simulink model "SerialPractice"  (R2017a)
settings: Solver=FixedStepAuto  StartTime=0.0  StopTime=10  FixedStep=0.01  MinStep=0.001  RelTol=1e-3  EnableMultiTasking=on
model workspace (MAT data in the model file):
  A = -0.291988550171
  B = -3.35930459163
  BladeGeometry = [0.0525  0.0575  0.0625  0.0675  0.0725  0.0775  0.0825  0.0875  0.0925  0.0975  0.1025  0.1075  0.1125  0.1175  0.1225  0.1275  0.1325  0.1375  0.1425  0.1475  0.1525  0.1575  0.1625  0.1675  0.1725  0.1775  0.1825  0.1875  0.1925  0.1975  0.2025  0.2075  0.2125  0.2175; 0.08  0.0765  0.073  0.0695  0.066  0.0625  0.059  0.0555  0.052  0.04975  0.0475  0.04525  0.043  0.0402857  0.0387143  0.0371429  0.0355714  0.034  0.0333333  0.0326667  0.032  0.0314615  0.0309231  0.0303846  0.0298462  0.0293077  0.0287692  0.0282308  0.0276923  0.0271538  0.0266154  0.0260769  0.0255385  0.025; 0.488692190558  0.471238898038  0.453785605519  0.436332312999  0.418879020479  0.401425727959  0.383972435439  0.366519142919  0.349065850399  0.331612557879  0.314159265359  0.296705972839  0.279252680319  0.270526034059  0.261799387799  0.244346095279  0.226892802759  0.209439510239  0.191986217719  0.174532925199  0.157079632679  0.157079632679  0.13962634016  0.13962634016  0.1308996939  0.12217304764  0.12217304764  0.10471975512  0.10471975512  0.10471975512  0.0872664625997  0.0872664625997  0.0698131700798  0.0698131700798]
  BladeRadius = Simulink.Parameter
  BladeRadius.Value = 0.225
  BladeRadius.Description = 'Radius of wind turbine blades'
  BladeRadius.DataType = 'auto'
  BladeRadius.DocUnits = 'm'
  BladeRadius.Dimensions = [1 1]
  C = 1
  CMCoeff = [0.0123861263102 0.0100869558121 0.00395957088672 0.0120046459416 0.000145393148265 0.000524554028707 -0.00381348366885 -0.000510658981973 -0.000304808013498 2.16037945221e-05 0.000267649913084 4.61550112894e-05 9.1778058191e-06 -1.06753844495e-05 -3.61000286217e-07]
  Cd = 0
  CpCoeff = [0.0934076671766 -0.182695655179 -0.0130608968061 0.168650159118 0.0246505329395 0.00117759523912 -0.0369025055261 -0.00816552386632 -0.000573147306806 5.84997495063e-05 0.00238658647448 0.000659038696901 -7.31985706862e-05 -3.57393703765e-05 -1.38327249044e-06]
  CpMat = [0.00867983704985 0.0104119952175 0.0123710198164 0.0145730804094 0.0170242990451 0.0197435241026 0.0227463845937 0.0260322039195 0.0296166959289 0.0335240991161 0.0377450541312 0.0423117873146 ... (4998 elements, 98x51)]
  CpMat_Iter4 = [-0.0174922752096 -0.0192000701213 -0.0208598028222 -0.0224761244863 -0.0240370794106 -0.0255486645229 -0.0270020954202 -0.0283906118498 -0.0297102509597 -0.0309617439636 -0.0321423489123 -0.0332305675829 ... (1116 elements, 31x36)]
  CqMat = [0.00950017223156 0.0100359591617 0.0106270141471 0.0112699479611 0.0119664102911 0.012716418653 0.0135224725245 0.0143788698852 0.0152889983241 0.0162542310373 0.017269427342 0.0183409321549 ... (4998 elements, 98x51)]
  CqMat_Iter4 = [-0.00651761334335 -0.00659827180546 -0.00666156814481 -0.00671038770655 -0.00674205252964 -0.00676213602267 -0.00676868409106 -0.00675993762446 -0.00673707609481 -0.00670413785211 -0.00666096044769 -0.00660422169135 ... (1116 elements, 31x36)]
  CtMat = [0.288467055178 0.29651503174 0.305320968805 0.314902207207 0.325269269239 0.336435131701 0.348427518089 0.36126603808 0.374993913486 0.389643061676 0.405260174906 0.421894254361 ... (4998 elements, 98x51)]
  CtMat_Iter4 = [0.270159937237 0.280676295654 0.292081972796 0.304364398908 0.317529220148 0.331577864133 0.346520948014 0.362369170866 0.379136481365 0.396833726898 0.415486832803 0.435128972133 ... (1116 elements, 31x36)]
  D = 0
  F = 8.99888284546
  G1 = 3.42440748358
  G2 = 0.707106781187
  GenPowerMat = [2.1012  1.98778255814  1.98947398844  1.833  1.70067971429  1.60026934097  1.384  1.0602; 9.4178  9.28771731844  8.83061432665  8.22752322946  7.62277971429  7.15313467049  6.01480738636  4.6053; 21.4169168091  21.0754826816  20.2670740984  18.7315883853  17.5955201987  16.3692763533  13.7873926136  10.6428; 35.1462940199  34.5144  33.2681888525  31.0593408638  29.3015798013  26.962289701  23.0149870432  17.6614; 48.8871910299  47.9983864865  49.251115894  45.8618066667  43.6489896806  40.1456687919  34.2611  26.4765; 67.2083870432  65.9770351351  70.6018443709  69.518850495  66.5248619165  60.9904074074  51.834  40.6659472362]
  GenTorqMat = [0.0501624549637  0.047454812988  0.0474951929119  0.0437596516031  0.0406007374717  0.0382036163842  0.0330405661859  0.0253104105999; 0.119911153844  0.118254889702  0.11243487365  0.10475607931  0.0970562457303  0.0910765393128  0.0765829061828  0.0586365007537; 0.185924082325  0.182960031645  0.17594209226  0.162612266372  0.152749855412  0.142104613443  0.11969081928  0.0923920488185; 0.239730275341  0.235420172908  0.226919858725  0.211853469756  0.199863911391  0.183907786414  0.156983526562  0.120467104797; 0.274610756692  0.269618134227  0.276655007553  0.257616466946  0.245186557716  0.225507587011  0.192452591321  0.148724677086; 0.305615629004  0.300016322066  0.321046643515  0.316121962696  0.302507446072  0.277340709145  0.235703923435  0.184919614804]
  J = Simulink.Parameter
  J.Value = 0.01
  J.Description = 'Resistance Inertia (0.2708)'
  J.DataType = 'auto'
  J.DocUnits = 'kg*m^2'
  J.Dimensions = [1 1]
  K = -0.496937064767
  Kd = Simulink.Parameter
  Kd.Value = 3
  Kd.DataType = 'auto'
  Kd.Dimensions = [1 1]
  Ki = Simulink.Parameter
  Ki.Value = 3
  Ki.DataType = 'auto'
  Ki.Dimensions = [1 1]
  Kp = Simulink.Parameter
  Kp.Value = 4
  Kp.DataType = 'auto'
  Kp.Dimensions = [1 1]
  Kt = Simulink.Parameter
  Kt.Value = 0.045
  Kt.Description = 'Torque Constant
    | '
  Kt.DataType = 'auto'
  Kt.DocUnits = 'N*m/A'
  Kt.Dimensions = [1 1]
  MaxPitch = Simulink.Parameter
  MaxPitch.Value = 30
  MaxPitch.Description = 'Maximum pitch angle'
  MaxPitch.DataType = 'auto'
  MaxPitch.DocUnits = 'deg'
  MaxPitch.Dimensions = [1 1]
  MaxPitchRate = Simulink.Parameter
  MaxPitchRate.Value = 150
  MaxPitchRate.Description = 'Maximum pitch rate'
  MaxPitchRate.DataType = 'auto'
  MaxPitchRate.DocUnits = 'deg/s'
  MaxPitchRate.Dimensions = [1 1]
  MinPitch = Simulink.Parameter
  MinPitch.Value = -10
  MinPitch.Description = 'Minimum pitch angle'
  MinPitch.DataType = 'auto'
  MinPitch.Dimensions = [1 1]
  N = Simulink.Parameter
  N.Value = 3
  N.Description = 'Number of blades'
  N.DataType = 'auto'
  N.Dimensions = [1 1]
  Ns = Simulink.Parameter
  Ns.Value = 2300
  Ns.Description = 'Generator sychronous speed'
  Ns.DataType = 'auto'
  Ns.DocUnits = 'rpm'
  Ns.Dimensions = [1 1]
  Param = Simulink.Parameter
  Param.DataType = 'auto'
  Param.Dimensions = [0 0]
  Param1 = Simulink.Parameter
  Param1.DataType = 'auto'
  Param1.Dimensions = [0 0]
  PowerMat = [0 0.000462072097155 0.000925921899779 0.00139155621296 0.00185898187069 0.00232820573596 0.00279923470096 0.00327207568717 0.00374673564551 0.00422322155651 0.00470154043042 0.00518169930735 ... (3512982 elements, 6666x17x31)]
  Qcog = Simulink.Parameter
  Qcog.Value = 0.01
  Qcog.Description = 'Cogging Torque'
  Qcog.DataType = 'auto'
  Qcog.DocUnits = 'N*m'
  Qcog.Dimensions = [1 1]
  R1 = Simulink.Parameter
  R1.Value = 4000
  R1.Description = 'Resistance of Divider_1'
  R1.DataType = 'auto'
  R1.DocUnits = 'Ω'
  R1.Dimensions = [1 1]
  R11 = Simulink.Parameter
  R11.Value = 1000
  R11.Description = 'Resistance of Divider_1.1'
  R11.DataType = 'auto'
  R11.DocUnits = 'Ω'
  R11.Dimensions = [1 1]
  R1_g = Simulink.Parameter
  R1_g.Value = 0.461
  R1_g.Description = 'A resistance in the generator'
  R1_g.DataType = 'auto'
  R1_g.DocUnits = 'Ω'
  R1_g.Dimensions = [1 1]
  R2 = Simulink.Parameter
  R2.Value = 4000
  R2.Description = 'Resistance of Divider_2'
  R2.DataType = 'auto'
  R2.DocUnits = 'Ω'
  R2.Dimensions = [1 1]
  R21 = Simulink.Parameter
  R21.Value = 1000
  R21.Description = 'Resistance of Divider_2.1'
  R21.DataType = 'auto'
  R21.DocUnits = 'Ω'
  R21.Dimensions = [1 1]
  R2_g = Simulink.Parameter
  R2_g.Value = 0.258
  R2_g.Description = 'A resistance in the generator'
  R2_g.DataType = 'auto'
  R2_g.DocUnits = 'Ω'
  R2_g.Dimensions = [1 1]
  R3 = Simulink.Parameter
  R3.Value = 4000
  R3.Description = 'Resistance of Divider_3'
  R3.DataType = 'auto'
  R3.DocUnits = 'Ω'
  R3.Dimensions = [1 1]
  R31 = Simulink.Parameter
  R31.Value = 1000
  R31.Description = 'Resistance of Divider_3.1'
  R31.DataType = 'auto'
  R31.DocUnits = 'Ω'
  R31.Dimensions = [1 1]
  R4 = Simulink.Parameter
  R4.Value = 4000
  R4.Description = 'Resistance of Divider_4'
  R4.DataType = 'auto'
  R4.DocUnits = 'Ω'
  R4.Dimensions = [1 1]
  R41 = Simulink.Parameter
  R41.Value = 1000
  R41.Description = 'Resistance of Divider_4.1'
  R41.DataType = 'auto'
  R41.DocUnits = 'Ω'
  R41.Dimensions = [1 1]
  RLoad = Simulink.Parameter
  RLoad.Value = 5
  RLoad.Description = 'Load Resistance'
  RLoad.DataType = 'auto'
  RLoad.DocUnits = 'Ω'
  RLoad.Dimensions = [1 1]
  RecEtaMat = [0.532050517794  0.556339856293  0.598601501945  0.632460223886  0.654778854328  0.673199910169  0.689313985989  0.719702240323; 0.640021818962  0.649839345247  0.704402948462  0.741053660874  0.759421104049  0.785862330559  0.801379702613  0.814844805672; 0.683186617187  0.690435804048  0.748352054458  0.784894367059  0.807903008646  0.82623891564  0.844204264611  0.864587511599; 0.705089035203  0.714712461207  0.767898438813  0.804077520567  0.825869089572  0.843984418142  0.863025059657  0.875924650746; 0.72694096499  0.738846952049  0.779691133184  0.813409899919  0.832582419359  0.854468949637  0.869961178839  0.889436670984; 0.756076871372  0.771026273171  0.795310298878  0.824051978599  0.837828909019  0.855066145496  0.873487304826  0.890319100762]
  Rs = Simulink.Parameter
  Rs.Value = 0.1
  Rs.Description = 'Shunt Resistor Resistance'
  Rs.DataType = 'auto'
  Rs.DocUnits = 'Ω'
  Rs.Dimensions = [1 1]
  TorqueMat = [0.0188980666332 0.0189200731218 0.0189421220361 0.0189642134844 0.0189863475752 0.0190085244173 0.01903074412 0.0190530067929 0.0190753125458 0.0190976614891 0.0191200537334 0.0191424893896 ... (3512982 elements, 6666x17x31)]
  V_r = 11
  X1 = Simulink.Parameter
  X1.Value = 0.507
  X1.Description = 'A resistance in the generator'
  X1.DataType = 'auto'
  X1.DocUnits = 'Ohm'
  X1.Dimensions = [1 1]
  X2 = Simulink.Parameter
  X2.Value = 0.309
  X2.Description = 'A resistance in the generator'
  X2.DataType = 'auto'
  X2.DocUnits = 'Ω'
  X2.Dimensions = [1 1]
  Xm = Simulink.Parameter
  Xm.Value = 30.74
  Xm.Description = 'A resistance in the generator'
  Xm.DataType = 'auto'
  Xm.DocUnits = 'Ω'
  Xm.Dimensions = [1 1]
  bladeRpmVec = [309.48670493 412.998876697 516.511048465 620.023220232 723.535392 827.047563768 930.559735535 1034.0719073 1137.58407907 1241.09625084 1344.60842261 1448.12059437 1551.63276614 1655.14493791 1758.65710968 1862.16928144 1965.68145321 2069.19362498 2172.70579675 2276.21796851 2379.73014028 2483.24231205 2586.75448382 2690.26665558 2793.77882735 2897.29099912 3000.80317089 3104.31534266 3207.82751442 3311.33968619 3414.85185796 3518.36402973 3621.87620149 3725.38837326 3828.90054503 3932.4127168 4035.92488856 4139.43706033 4242.9492321 4346.46140387 4449.97357563]
  bladeTorqueMat = [0.00679712928319 0.00852288742527 0.0105717008499 0.012902132331 0.0154507893622 0.0182235621523 0.021227269718 0.0244699016413 0.0279609406934 0.0317118022158 0.0409095986767 0.0494145988338 ... (656 elements, 16x41)]
  break1_Cp = [1 2 3 4 5 6 7 8 9 10 11 12 13 14 15 16 17 18 19 20 21 22 23 24 25 26 27 28 29 30 31 32 33 34 35 36 37 38 39 40 41 42 43 44 45 46 47 48 49 50 51 52 53 54 55 56 57 58 59 60 61 62 63 64 65 66 67 68 69 70 71 72 73 74 75 76 77 78 79 80 81 82 83 84 85 86 87 88 89 90 91 92 93 94 95 96 97 98]
  break1_Cp_Iter4 = [1 1.1 1.2 1.3 1.4 1.5 1.6 1.7 1.8 1.9 2 2.1 2.2 2.3 2.4 2.5 2.6 2.7 2.8 2.9 3 3.1 3.2 3.3 3.4 3.5 3.6 3.7 3.8 3.9 4]
  break1_Cq = [1 2 3 4 5 6 7 8 9 10 11 12 13 14 15 16 17 18 19 20 21 22 23 24 25 26 27 28 29 30 31 32 33 34 35 36 37 38 39 40 41 42 43 44 45 46 47 48 49 50 51 52 53 54 55 56 57 58 59 60 61 62 63 64 65 66 67 68 69 70 71 72 73 74 75 76 77 78 79 80 81 82 83 84 85 86 87 88 89 90 91 92 93 94 95 96 97 98]
  break1_Cq_Iter4 = [1 1.1 1.2 1.3 1.4 1.5 1.6 1.7 1.8 1.9 2 2.1 2.2 2.3 2.4 2.5 2.6 2.7 2.8 2.9 3 3.1 3.2 3.3 3.4 3.5 3.6 3.7 3.8 3.9 4]
  break1_Ct = [1 2 3 4 5 6 7 8 9 10 11 12 13 14 15 16 17 18 19 20 21 22 23 24 25 26 27 28 29 30 31 32 33 34 35 36 37 38 39 40 41 42 43 44 45 46 47 48 49 50 51 52 53 54 55 56 57 58 59 60 61 62 63 64 65 66 67 68 69 70 71 72 73 74 75 76 77 78 79 80 81 82 83 84 85 86 87 88 89 90 91 92 93 94 95 96 97 98]
  break1_Ct_Iter4 = [1 1.1 1.2 1.3 1.4 1.5 1.6 1.7 1.8 1.9 2 2.1 2.2 2.3 2.4 2.5 2.6 2.7 2.8 2.9 3 3.1 3.2 3.3 3.4 3.5 3.6 3.7 3.8 3.9 4]
  break2_Cp = [1 2 3 4 5 6 7 8 9 10 11 12 13 14 15 16 17 18 19 20 21 22 23 24 25 26 27 28 29 30 31 32 33 34 35 36 37 38 39 40 41 42 43 44 45 46 47 48 49 50 51]
  break2_Cp_Iter4 = [-15 -14 -13 -12 -11 -10 -9 -8 -7 -6 -5 -4 -3 -2 -1 0 1 2 3 4 5 6 7 8 9 10 11 12 13 14 15 16 17 18 19 20]
  break2_Cq = [1 2 3 4 5 6 7 8 9 10 11 12 13 14 15 16 17 18 19 20 21 22 23 24 25 26 27 28 29 30 31 32 33 34 35 36 37 38 39 40 41 42 43 44 45 46 47 48 49 50 51]
  break2_Cq_Iter4 = [-15 -14 -13 -12 -11 -10 -9 -8 -7 -6 -5 -4 -3 -2 -1 0 1 2 3 4 5 6 7 8 9 10 11 12 13 14 15 16 17 18 19 20]
  break2_Ct = [1 2 3 4 5 6 7 8 9 10 11 12 13 14 15 16 17 18 19 20 21 22 23 24 25 26 27 28 29 30 31 32 33 34 35 36 37 38 39 40 41 42 43 44 45 46 47 48 49 50 51]
  break2_Ct_Iter4 = [-15 -14 -13 -12 -11 -10 -9 -8 -7 -6 -5 -4 -3 -2 -1 0 1 2 3 4 5 6 7 8 9 10 11 12 13 14 15 16 17 18 19 20]
  dpitch = 1
  dpitch_Iter4 = 1
  dtsr = 0.1
  dtsr_Iter4 = 0.1
  generatorRpmVec = [341.915107735; 356.773957938; 376.905565127; 404.234314097; 443.280769879; 505.264428386; 745.052922197; 1327.1653456; 1680.18103026; 1897.48535507; 2373.16400484; 2811.26212639; 3191.52215225; 3554.79100153; 3921.64355962; 4336.45118522]
  generatorTorqueVec = [0.0238551497253; 0.030635265767; 0.0389685483873; 0.0489528429291; 0.0610820639797; 0.076486398825; 0.111942081576; 0.216661798023; 0.273917248647; 0.300910880899; 0.342762941842; 0.368779609659; 0.385571161846; 0.398257187871; 0.40868321227; 0.418347136739]
  loadBreak = [0.5 0.6 0.8 1 1.3 1.5 2 3]
  n = Simulink.Parameter
  n.Value = 34
  n.Description = 'Number of blade segments'
  n.DataType = 'auto'
  n.Dimensions = [1 1]
  oBreak = [0 0.103201266712 0.206402533425 0.309603800137 0.412805066849 0.516006333561 0.619207600274 0.722408866986 0.825610133698 0.928811400411 1.03201266712 1.13521393384 ... (6666 elements, 1x6666)]
  omega_initial = Simulink.Parameter
  omega_initial.Value = 104.71975512
  omega_initial.Description = 'Initial angular velocity of turbine'
  omega_initial.DataType = 'auto'
  omega_initial.DocUnits = 'rad/s'
  omega_initial.Dimensions = [1 1]
  p = -2.67879336333
  pBreak = [0 1 2 3 4 5 6 7 8 9 10 11 12 13 14 15 16 17 18 19 20 21 22 23 24 25 26 27 28 29 30]
  pitchIn = [-10; -9; -8; -7; -6; -5; -4; -3; -2; -1; 0; 1; 2; 3; 4; 5; 6; 7; 8; 9; 10; 11; 12; 13; 14; 15; 16; 17; 18; 19; 20; 21; 22; 23; 24; 25; 26; 27; 28; 29; 30]
  pitchMat = [-10 -10 -10 -10 -10 -10 -10 -10 -10 -10 -10 -10 ... (656 elements, 16x41)]
  pitchMat_Cp = [1 1 1 1 1 1 1 1 1 1 1 1 ... (4998 elements, 98x51)]
  pitchMat_Cp_Iter4 = [-15 -14 -13 -12 -11 -10 -9 -8 -7 -6 -5 -4 -3 -2 -1 0 1 2 3 4 5 6 7 8 9 10 11 12 13 14 15 16 17 18 19 20]
  pitchMat_Cq = [1 1 1 1 1 1 1 1 1 1 1 1 ... (4998 elements, 98x51)]
  pitchMat_Cq_Iter4 = [-15 -14 -13 -12 -11 -10 -9 -8 -7 -6 -5 -4 ... (1116 elements, 36x31)]
  pitchMat_Ct = [1 1 1 1 1 1 1 1 1 1 1 1 ... (4998 elements, 98x51)]
  pitchMat_Ct_Iter4 = [-15 -14 -13 -12 -11 -10 -9 -8 -7 -6 -5 -4 ... (1116 elements, 36x31)]
  pitchOpt = [13; 14; 13; 9; 7; 4; 3; 10; 15; 18; 21; 23; 27; 28; 29; 30]
  pitchVec = [-10; -9; -8; -7; -6; -5; -4; -3; -2; -1; 0; 1; 2; 3; 4; 5; 6; 7; 8; 9; 10; 11; 12; 13; 14; 15; 16; 17; 18; 19; 20; 21; 22; 23; 24; 25; 26; 27; 28; 29; 30]
  pitch_Start = -10
  pitch_Start_Iter4 = -15
  pitch_r = 11
  rho = Simulink.Parameter
  rho.Value = 1.225
  rho.Description = 'Density of air'
  rho.DataType = 'auto'
  rho.DocUnits = 'kg/m^3'
  rho.Dimensions = [1 1]
  rpmBreak = [400 750 1100 1400 1700 2100]
  rpmMat = [309.48670493 312.485093221 316.121075916 320.361079054 325.130275341 330.482781466 336.483551241 343.211141152 350.761447592 359.252856498 381.925342476 405.594424779 ... (656 elements, 16x41)]
  tsrMat_Cp = [1 1.1 1.2 1.3 1.4 1.5 1.6 1.7 1.8 1.9 2 2.1 ... (4998 elements, 98x51)]
  tsrMat_Cp_Iter4 = [1 1.1 1.2 1.3 1.4 1.5 1.6 1.7 1.8 1.9 2 2.1 2.2 2.3 2.4 2.5 2.6 2.7 2.8 2.9 3 3.1 3.2 3.3 3.4 3.5 3.6 3.7 3.8 3.9 4]
  tsrMat_Cq = [1 1.1 1.2 1.3 1.4 1.5 1.6 1.7 1.8 1.9 2 2.1 ... (4998 elements, 98x51)]
  tsrMat_Cq_Iter4 = [1 1 1 1 1 1 1 1 1 1 1 1 ... (1116 elements, 36x31)]
  tsrMat_Ct = [1 1.1 1.2 1.3 1.4 1.5 1.6 1.7 1.8 1.9 2 2.1 ... (4998 elements, 98x51)]
  tsrMat_Ct_Iter4 = [1 1 1 1 1 1 1 1 1 1 1 1 ... (1116 elements, 36x31)]
  tsrMin = Simulink.Parameter
  tsrMin.Value = 0.6
  tsrMin.Description = 'Minimum tip speed ratio for induced velcoity to take effect'
  tsrMin.DataType = 'auto'
  tsrMin.Dimensions = [1 1]
  tsr_Start = 1
  tsr_Start_Iter4 = 1
  vBreak = [5 6 7 8 9 10 11 12 13 14 15 16 17 18 19 20 21]
  windIn = [5; 6; 7; 8; 9; 10; 11; 12; 13; 14; 15; 16; 17; 18; 19; 20]
  windMat = [5 6 7 8 9 10 11 12 13 14 15 16 ... (656 elements, 16x41)]
  windVec = [5; 6; 7; 8; 9; 10; 11; 12; 13; 14; 15; 16; 17; 18; 19; 20]
undefined symbols (not defined in the file or in its folder): LF, serial
libraries: instrumentlib x3
(part 1 of 2)
settings Model: ExtModeTrigDuration=2  Creator=alexandermclean  LastModifiedBy=alexandermclean
settings OptimizationCC: PassReuseOutputArgsThreshold=0  MaxStackSize=64
settings DebuggingCC: ParameterTunabilityLossMsg=error
settings HardwareCC: ProdBitPerInt=16  ProdBitPerPointer=16  ProdBitPerSizeT=16  ProdBitPerPtrDiffT=16  ProdLargestAtomicFloat=None  ProdWordSize=8  ProdHWDeviceType=Atmel->AVR  UseEmbeddedCoderFeatures=off  UseSimulinkCoderFeatures=off
settings SFSimCC: MATLABDynamicMemAlloc=off
settings RTWCC: SystemTargetFile=ert.tlc  HardwareBoard=Arduino Uno  TLCOptions=-aInlineSetEventsForThisBaseRateFcn=TLC_FALSE -aSuppressMultiTaskScheduler=TLC_FALSE -aRateBasedStepFcn=1  TemplateMakefile=ert_default_tmf  Description=Embedded Coder  GenerateReport=on  Toolchain=Arduino AVR  BuildConfiguration=Faster Runs  LaunchReport=on
settings CodeAppCC: ForceParamTrailComments=on  OperatorAnnotations=on  MATLABSourceComments=on
settings ERTTargetCC: ERTCustomFileBanners=off  SupportContinuousTime=on  SupportNonInlinedSFcns=on  RemoveResetFunc=off  SupportVariableSizeSignals=on  ModelStepFunctionPrototypeControlCompliant=off

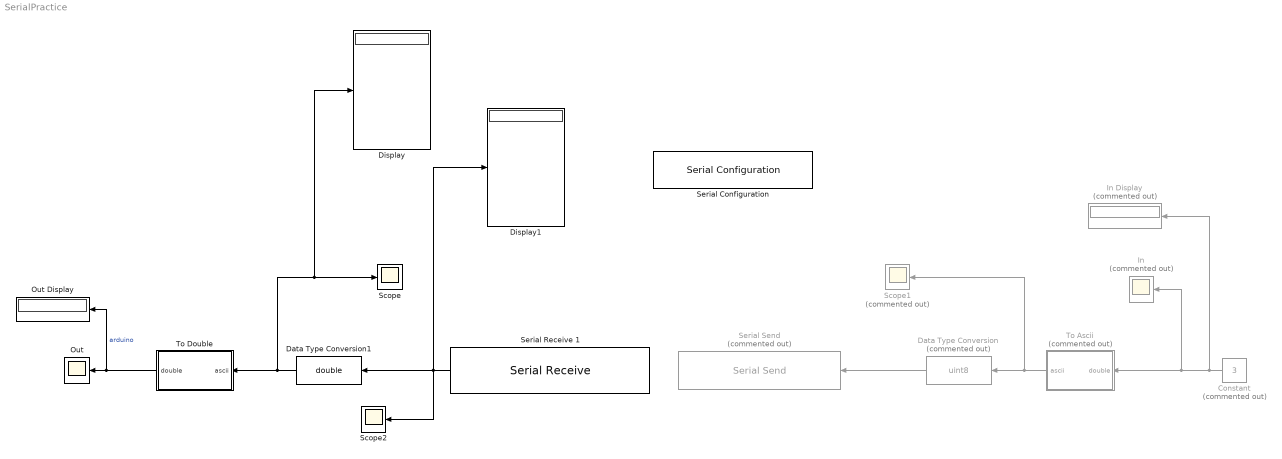
== system SerialPractice ==
  Constant : Constant  Commented=on  Value=3
  Data Type Conversion : DataTypeConversion  Commented=on  OutDataTypeStr=uint8
  Data Type Conversion1 : DataTypeConversion  OutDataTypeStr=double
  Display : Display
  Display1 : Display
  In : Scope  Commented=on
  In Display : Display  Commented=on
  Out : Scope
  Out Display : Display
  Scope : Scope
  Scope1 : Scope  Commented=on
  Scope2 : Scope
  Serial Configuration : instrumentlib/Serial Configuration  Priority=-200000  ComPortMenu=/dev/cu.Bluetooth-Incoming-Port  ComPort=/dev/cu.Bluetooth-Incoming-Port  ObjConstructor=serial('/dev/cu.Bluetooth-Incoming-Port');  BaudRate=9600  DataBits=8  Parity=none  StopBits=1  FlowControl=none  Timeout=10  ByteOrder=LittleEndian
  Serial Receive 1 : instrumentlib/Serial Receive  ComPortMenu=/dev/cu.Bluetooth-Incoming-Port  ComPort=/dev/cu.Bluetooth-Incoming-Port  ObjConstructor=serial('/dev/cu.Bluetooth-Incoming-Port');  Terminator=<none>  DataSize="[6  1]"  DataType=uint8  EnableBlockingMode=on  ActionDataUnavailable=Output last received value  CustomValue=0  SampleTime=0.01
  Serial Send : instrumentlib/Serial Send  Commented=on  ComPortMenu=<Please select a port...>  ComPort=/dev/cu.usbmodem1421  ObjConstructor=serial('/dev/cu.usbmodem1421');  Header=r  Terminator=LF ('\n')  EnableBlockingMode=on
  To Ascii : MATLAB Function  Commented=on  TreatAsAtomicUnit=on
  To Double : MATLAB Function  TreatAsAtomicUnit=on
  wires:
  Data Type Conversion/1 -> Serial Send/1
  To Ascii/1(ascii) -> Scope1/1, Data Type Conversion/1
  Data Type Conversion1/1 -> Display/1, Scope/1, To Double/1(ascii)
  Constant/1 -> In Display/1, To Ascii/1(double), In/1
  To Double/1(double) -> Out Display/1, Out/1  "arduino"
  Serial Receive 1/1 -> Display1/1, Scope2/1, Data Type Conversion1/1
